simulink model "BaseEngineController_A02_bak2"  (Simulink 7.7)
settings: Solver=FixedStepDiscrete  StartTime=0.0  StopTime=10.0  RelTol=1e-3
PreLoadFcn:
  | try, eval([bdroot '_setup']); catch, eval('model_setup', ''); end
InitFcn:
  | try, eval([bdroot '_setup']); catch, eval('model_setup', ''); end
undefined symbols (not defined in the file or in its folder): ECU555, High, motohawk_build_version, motohawk_trunc_name, motohawk_vardec_config_group, motohawk_vardec_path, True
libraries: MotoHawk_lib x1081, AutoDoc_lib x609, motohawk_control_primitives_lib x132, motohawk_engine_lib x26, motohawk_annotations_lib x9, simulink x8, motohawk_control_primitives_motoshare_lib x1, simulink_extras x1
(part 4 of 22)

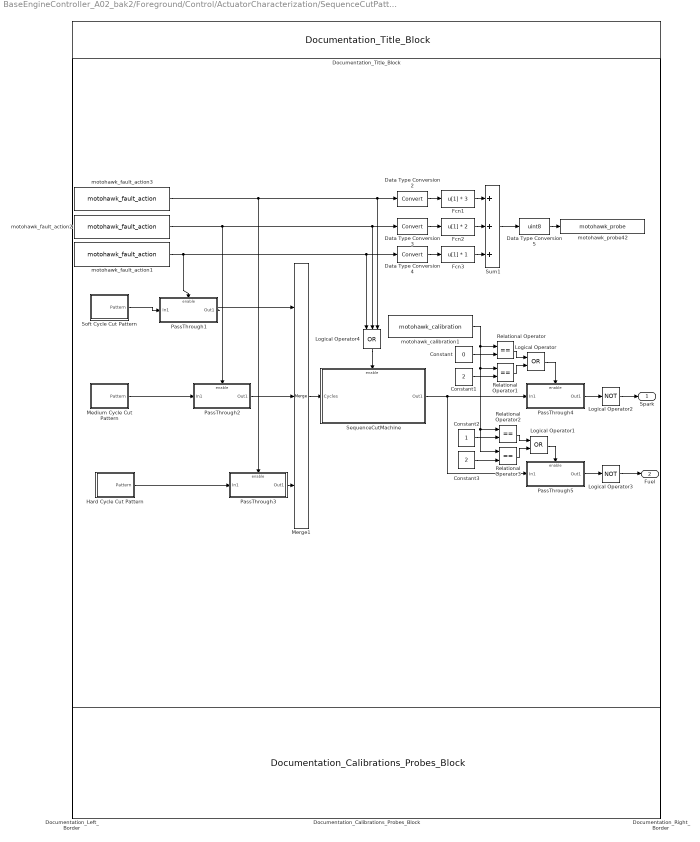
== system BaseEngineController_A02_bak2/Foreground/Control/ActuatorCharacterization/SequenceCutPattern ==
  Constant : Constant  Value=0  OutDataTypeStr=Inherit: Inherit via back propagation  SampleTime=-1
  Constant1 : Constant  Value=2  OutDataTypeStr=Inherit: Inherit via back propagation  SampleTime=-1
  Constant2 : Constant  OutDataTypeStr=Inherit: Inherit via back propagation  SampleTime=-1
  Constant3 : Constant  Value=2  OutDataTypeStr=Inherit: Inherit via back propagation  SampleTime=-1
  Data Type Conversion2 : DataTypeConversion  SaturateOnIntegerOverflow=on  RndMeth=Zero
  Data Type Conversion3 : DataTypeConversion  SaturateOnIntegerOverflow=on  RndMeth=Zero
  Data Type Conversion4 : DataTypeConversion  SaturateOnIntegerOverflow=on  RndMeth=Zero
  Data Type Conversion5 : DataTypeConversion  OutDataTypeStr=uint8  SaturateOnIntegerOverflow=on  RndMeth=Zero
  Documentation_Calibrations_Probes_Block : AutoDoc_lib/Documentation_Calibrations_Probes_Block
  Documentation_Left_Border : AutoDoc_lib/Documentation_Left_Border  layout=Portrait
  Documentation_Right_Border : AutoDoc_lib/Documentation_Right_Border  layout=Portrait
  Documentation_Title_Block : AutoDoc_lib/Documentation_Title_Block
  Fcn1 : Fcn  Expr=u[1] * 3
  Fcn2 : Fcn  Expr=u[1] * 2
  Fcn3 : Fcn  Expr=u[1] * 1
  Hard Cycle Cut Pattern : Subsystem
  Logical Operator : Logic  Operator=OR
  Logical Operator1 : Logic  Operator=OR
  Logical Operator2 : Logic  Operator=NOT
  Logical Operator3 : Logic  Operator=NOT
  Logical Operator4 : Logic  Operator=OR  Inputs=3  AllPortsSameDT=off
  Medium Cycle Cut Pattern : Subsystem
  Merge1 : Merge  Inputs=3  AllowUnequalInputPortWidths=on
  PassThrough1 : Subsystem  TreatAsAtomicUnit=on
  PassThrough2 : Subsystem  TreatAsAtomicUnit=on
  PassThrough3 : Subsystem  TreatAsAtomicUnit=on
  PassThrough4 : Subsystem  TreatAsAtomicUnit=on
  PassThrough5 : Subsystem  TreatAsAtomicUnit=on
  Relational Operator{,1..3} (4 alike) : RelationalOperator  Operator===  InputSameDT=on
  SequenceCutMachine : Subsystem  TreatAsAtomicUnit=on
      mask Description:
      | When enabled, this block cycles though the 4 input patterns of cylinder disables that can be applied to fuel or spark.
      | 
      | The output should act on the enable inputs for MotoHawk EST and FInj blocks.  Outputs are reset when disabled.
      | 
      | Calibration arrays should be cast as boolean, and can be set up for any cylinder configuration.
      mask Display:
      | patch([0 0 1 1], [0 0.075 0.075 0], [230/255 0/255 0/255])
      | patch([0 0 1 1], [0.025 0.05 0.05 0.025], [150/255 0/255 0/255])
      | patch([0 0 1 1], [0 0.025 0.025 0], [0/255 0/255 0/255])
      | 
      | disp('SEQUENCE DISABLE\nPATTERN');
  Soft Cycle Cut Pattern : Subsystem
  Sum1 : Sum  Inputs=+++  SaturateOnIntegerOverflow=on
  motohawk_calibration1 : MotoHawk_lib/Calibration & Probing Blocks/motohawk_calibration  nam='RevLimitType'  val=0  storage=Calibration  show_more=on  name_source=Use Parameter  data_type=int8  read_level=1  write_level=1  ovr_cals=on  view_as=Enumeration  enum_struct={'Cut Spark', 'Cut Fuel', 'Cut Spark and Fuel'}  vector_style=Wide Row  use_help_units=on  help='Define the type of sequence to be disable when in Rev Limit'  units=''  use_header_enum=off  row_header_enum={}  col_header_enum={}  use_min_max=off  min_val=-inf  max_val=inf  use_precision=off  precision=''  gain=1  offset=0  exp=1  use_mototune_group=on  mototune_group=motohawk_vardec_path(gcb)
  motohawk_fault_action1 : MotoHawk_lib/Fault Management Blocks/motohawk_fault_action  nam='SoftRevLimit'  output_status=on  eval_nam=SoftRevLimit
  motohawk_fault_action2 : MotoHawk_lib/Fault Management Blocks/motohawk_fault_action  nam='MediumRevLimit'  output_status=on  eval_nam=MediumRevLimit
  motohawk_fault_action3 : MotoHawk_lib/Fault Management Blocks/motohawk_fault_action  nam='HardRevLimit'  output_status=on  eval_nam=HardRevLimit
  motohawk_probe42 : MotoHawk_lib/Calibration & Probing Blocks/motohawk_probe  nam='RevLimiterState'  show_more=on  name_source=Use Parameter  read_level=1  view_as=Enumeration  enum_struct={'Disabled', 'Soft', 'Medium', 'Hard'}  vector_style=Wide Row  use_help_units=off  help=''  units=''  use_header_enum=off  row_header_enum={}  col_header_enum={}  use_precision=off  precision=''  gain=1  offset=0  exp=1  use_mototune_group=on  mototune_group=motohawk_vardec_path(gcb)
  Spark : Outport
  Fuel : Outport  Port=2
  wires:
  Logical Operator/1 -> PassThrough4/Enable
  PassThrough4/1(Out1) -> Logical Operator2/1
  PassThrough5/1(Out1) -> Logical Operator3/1
  Logical Operator1/1 -> PassThrough5/Enable
  Relational Operator/1 -> Logical Operator/1
  Relational Operator1/1 -> Logical Operator/2
  motohawk_calibration1/1 -> Relational Operator/1, Relational Operator1/1, Relational Operator2/1, Relational Operator3/1
  Constant/1 -> Relational Operator/2
  Constant1/1 -> Relational Operator1/2
  Constant2/1 -> Relational Operator2/2
  Constant3/1 -> Relational Operator3/2
  Relational Operator2/1 -> Logical Operator1/1
  Relational Operator3/1 -> Logical Operator1/2
  Logical Operator2/1 -> Spark/1
  Logical Operator3/1 -> Fuel/1
  SequenceCutMachine/1(Out1) -> PassThrough4/1(In1), PassThrough5/1(In1)
  Merge1/1 -> SequenceCutMachine/1(Cycles)
  PassThrough1/1(Out1) -> Merge1/1
  motohawk_fault_action1/1 -> PassThrough1/Enable, Logical Operator4/1, Data Type Conversion4/1
  Medium Cycle Cut Pattern/1(Pattern) -> PassThrough2/1(In1)
  PassThrough2/1(Out1) -> Merge1/2
  motohawk_fault_action2/1 -> PassThrough2/Enable, Logical Operator4/2, Data Type Conversion3/1
  Hard Cycle Cut Pattern/1(Pattern) -> PassThrough3/1(In1)
  PassThrough3/1(Out1) -> Merge1/3
  motohawk_fault_action3/1 -> PassThrough3/Enable, Logical Operator4/3, Data Type Conversion2/1
  Logical Operator4/1 -> SequenceCutMachine/Enable
  Soft Cycle Cut Pattern/1(Pattern) -> PassThrough1/1(In1)
  Data Type Conversion2/1 -> Fcn1/1
  Data Type Conversion3/1 -> Fcn2/1
  Data Type Conversion4/1 -> Fcn3/1
  Fcn1/1 -> Sum1/1
  Fcn2/1 -> Sum1/2
  Fcn3/1 -> Sum1/3
  Sum1/1 -> Data Type Conversion5/1
  Data Type Conversion5/1 -> motohawk_probe42/1

== system BaseEngineController_A02_bak2/Foreground/Control/ActuatorCharacterization/Spark Advance to Start Angle ==
  Stop Angle [degCA] : Inport
  RPM : Inport  Port=2
  PulseWidth [ms] : Inport  Port=3
  Trigger : TriggerPort  TriggerType=function-call
  Documentation_Calibrations_Probes_Block : AutoDoc_lib/Documentation_Calibrations_Probes_Block
  Documentation_Left_Border : AutoDoc_lib/Documentation_Left_Border  layout=Portrait
  Documentation_Right_Border : AutoDoc_lib/Documentation_Right_Border  layout=Portrait
  Documentation_Title_Block : AutoDoc_lib/Documentation_Title_Block
  Product1 : Product  Inputs=**/  SaturateOnIntegerOverflow=on  InputSameDT=on  OutDataTypeStr=Inherit: Same as first input
  Product2 : Product  Inputs=**  SaturateOnIntegerOverflow=on  InputSameDT=on  OutDataTypeStr=Inherit: Same as first input
  Sum : Sum  Inputs=|++  SaturateOnIntegerOverflow=on  InputSameDT=on
  deg/rev : Constant  Value=360
  min/msec : Constant  Value=60000
  Start Angle [degCA] : Outport
  wires:
  Sum/1 -> Start Angle [degCA]/1
  RPM/1 -> Product1/1
  min/msec/1 -> Product1/3
  deg/rev/1 -> Product1/2
  PulseWidth [ms]/1 -> Product2/2  "PulseWidth [ms]"
  Product1/1 -> Product2/1  "deg/ms"
  Product2/1 -> Sum/2  "PulseWidth [degCA]"
  Stop Angle [degCA]/1 -> Sum/1

== system BaseEngineController_A02_bak2/Foreground/Control/ActuatorCharacterization/Spark Coil Characterization ==
  SparkEnergy [mJ] : Inport
  SysVolt09Idx : Inport  Port=2
  Trigger : TriggerPort  TriggerType=function-call
  Constant : Constant  Value=1000
  Constant1 : Constant  Value=0  OutDataTypeStr=Inherit: Inherit via back propagation
  Documentation_Calibrations_Probes_Block : AutoDoc_lib/Documentation_Calibrations_Probes_Block
  Documentation_Left_Border : AutoDoc_lib/Documentation_Left_Border  layout=Portrait
  Documentation_Right_Border : AutoDoc_lib/Documentation_Right_Border  layout=Portrait
  Documentation_Title_Block : AutoDoc_lib/Documentation_Title_Block
  Only Enable Spark when Energy is Above Zero : RelationalOperator  Operator=>  ZeroCross=off  InputSameDT=on
  Product : Product  Inputs=*/  SaturateOnIntegerOverflow=on  OutDataTypeStr=Inherit: Same as first input
  Sum : Sum  Inputs=|++  SaturateOnIntegerOverflow=on
  motohawk_calibration : MotoHawk_lib/Calibration & Probing Blocks/motohawk_calibration  nam='CoilDrvrDlyTime'  val=0.004  storage=Calibration  show_more=on  name_source=Use Parameter  data_type=Inherit from 'Default Value'  read_level=1  write_level=1  ovr_cals=on  view_as=Number  enum_struct={'Disabled', 'Enabled'}  vector_style=Wide Row  use_help_units=on  help='Coil driver delay time between receipt of EST signal and initiation of coil dwell.'  units='ms'  use_header_enum=off  row_header_enum={}  col_header_enum={}  use_min_max=off  min_val=-inf  max_val=inf  use_precision=off  precision='2.3'  gain=1  offset=0  exp=1  use_mototune_group=on  mototune_group=motohawk_vardec_path(gcb)
  motohawk_interpolation_2d1 : MotoHawk_lib/Lookup Tables/motohawk_interpolation_2d  nam='CoilDwell'  table_data=ones(9)*2  show_more=on  data_type=Inherit from 'Table Data'  storage=NonAdaptive  read_level=1  write_level=1  ovr_cals=on  use_help=on  help='Required spark dwell to give the desired spark energy.'  units='ms'  use_min_max=on  min_val=-inf  max_val=inf  use_precision=on  precision=''  gain=1  offset=0  exp=1  use_links=off  row_prelookup_block=''  col_prelookup_block=''  output_name=''  use_mototune_group=on  mototune_group=motohawk_vardec_path(gcb)
  motohawk_override_rel : MotoHawk_lib/Calibration & Probing Blocks/motohawk_override_rel  SystemSampleTime=-1  parent_name='SparkDwell'  show_more=on  name_source=Use Parameter  parent_read_level=1  parent_override_level=1  use_help_units=off  parent_help=''  parent_units=''  use_min_max=off  parent_min=-inf  parent_max=inf  use_precision=off  parent_precision=''  parent_gain=1  parent_offset=0  parent_exp=1  use_mototune_group=on  parent_group=motohawk_vardec_path(gcb)
  motohawk_prelookup : MotoHawk_lib/Lookup Tables/motohawk_prelookup  nam='SpkEnergy09'  breakpoint_data=[10 19 29 38 48 57 66 76 86]  show_more=on  data_type=Inherit from 'Table Data'  storage=Constant  read_level=1  write_level=1  ovr_cals=on  use_help=on  help=''  units=''  use_min_max=off  min_val=-inf  max_val=inf  use_precision=on  precision=''  gain=1  offset=0  exp=1  use_links=off  input_name=''  use_mototune_group=on  mototune_group=motohawk_vardec_path(gcb)
  motohawk_probe : MotoHawk_lib/Calibration & Probing Blocks/motohawk_probe  nam='SparkDwell'  show_more=on  name_source=Use Parameter  read_level=1  view_as=Number  enum_struct={'Disabled', 'Enabled'}  vector_style=Wide Row  use_help_units=on  help='Total electrical spark dwell time'  units='ms'  use_header_enum=off  row_header_enum={}  col_header_enum={}  use_precision=off  precision=''  gain=1  offset=0  exp=1  use_mototune_group=on  mototune_group=motohawk_vardec_path(gcb)
  motohawk_probe1 : MotoHawk_lib/Calibration & Probing Blocks/motohawk_probe  nam='SparkEnergyMonitor'  show_more=on  name_source=Use Parameter  read_level=1  view_as=Number  enum_struct={'Disabled', 'Enabled'}  vector_style=Wide Row  use_help_units=on  help='Commanded spark energy, before conversion to dwell time'  units='mJ'  use_header_enum=off  row_header_enum={}  col_header_enum={}  use_precision=off  precision=''  gain=1  offset=0  exp=1  use_mototune_group=on  mototune_group=motohawk_vardec_path(gcb)
  motohawk_probe2 : MotoHawk_lib/Calibration & Probing Blocks/motohawk_probe  nam='SparkEnabled'  show_more=on  name_source=Use Parameter  read_level=1  view_as=Number  enum_struct={'Disabled', 'Enabled'}  vector_style=Wide Row  use_help_units=on  help='Enabled when spark energy is greater than zero'  units='boolean'  use_header_enum=off  row_header_enum={}  col_header_enum={}  use_precision=off  precision=''  gain=1  offset=0  exp=1  use_mototune_group=on  mototune_group=motohawk_vardec_path(gcb)
  SparkDwell [ms] : Outport
  SparkEnable [bool] : Outport  Port=2
  wires:
  SysVolt09Idx/1 -> motohawk_interpolation_2d1/2  "SysVolt10Idx"
  motohawk_prelookup/1 -> motohawk_interpolation_2d1/1  "SpkEnergy09Idx"
  SparkEnergy [mJ]/1 -> motohawk_probe1/1, Only Enable Spark when Energy is Above Zero/1, motohawk_prelookup/1
  Sum/1 -> motohawk_override_rel/1
  motohawk_calibration/1 -> Product/1
  Product/1 -> Sum/2
  Constant/1 -> Product/2
  Only Enable Spark when Energy is Above Zero/1 -> SparkEnable [bool]/1, motohawk_probe2/1
  Constant1/1 -> Only Enable Spark when Energy is Above Zero/2
  motohawk_override_rel/1 -> motohawk_probe/1, SparkDwell [ms]/1
  motohawk_interpolation_2d1/1 -> Sum/1

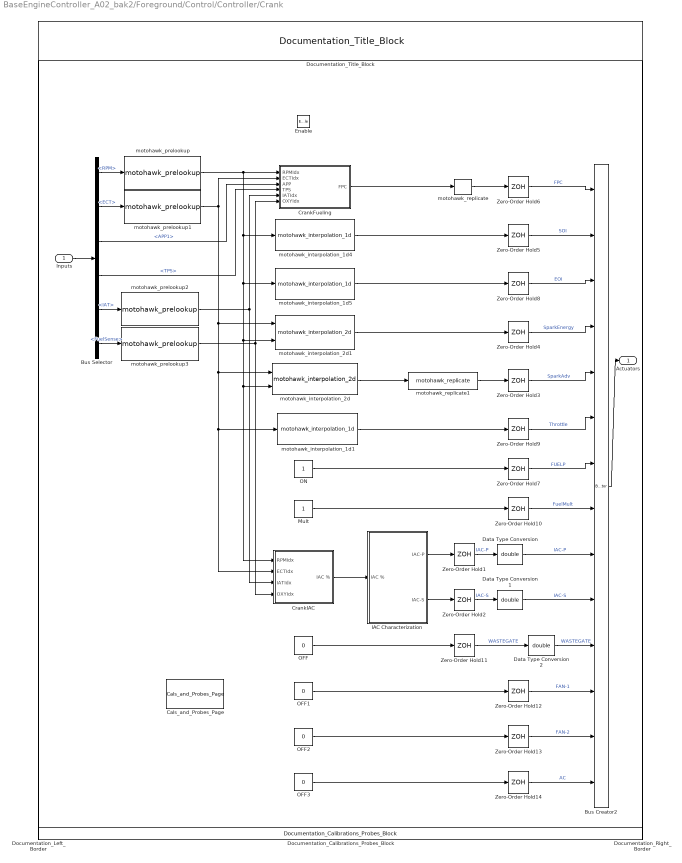
== system BaseEngineController_A02_bak2/Foreground/Control/Controller/Crank ==
  Inputs : Inport
  Enable : EnablePort  StatesWhenEnabling=reset
  Bus Creator2 : BusCreator  Inputs=14
  Bus Selector : BusSelector  OutputSignals=Raw.RPM,Raw.NonLinearSensors.ECT,RawProcessed.APP1,RawProcessed.TPS,Raw.NonLinearSensors.IAT,Raw.LinearSensors.FuelSense
  Cals_and_Probes_Page : AutoDoc_lib/Cals_and_Probes_Page  SystemSampleTime=-1
  CrankFueling : Subsystem
  CrankIAC : Subsystem
  Data Type Conversion : DataTypeConversion  OutDataTypeStr=double
  Data Type Conversion1 : DataTypeConversion  OutDataTypeStr=double
  Data Type Conversion2 : DataTypeConversion  OutDataTypeStr=double
  Documentation_Calibrations_Probes_Block : AutoDoc_lib/Documentation_Calibrations_Probes_Block
  Documentation_Left_Border : AutoDoc_lib/Documentation_Left_Border  layout=Portrait
  Documentation_Right_Border : AutoDoc_lib/Documentation_Right_Border  layout=Portrait
  Documentation_Title_Block : AutoDoc_lib/Documentation_Title_Block
  IAC Characterization : Subsystem
  Mult : Constant  OutDataTypeStr=double
  OFF{,1..3} (4 alike) : Constant  Value=0  OutDataTypeStr=boolean
  ON : Constant  OutDataTypeStr=boolean
  Zero-Order Hold{1..14} (14 alike) : ZeroOrderHold  SampleTime=-1
  motohawk_interpolation_1d1 : MotoHawk_lib/Lookup Tables/motohawk_interpolation_1d  nam='CrankAirflowSetPt'  table_data=[34 34 34 31 30]  show_more=on  data_type=Inherit from 'Table Data'  storage=NonAdaptive  read_level=1  write_level=1  ovr_cals=on  use_help=on  help='ETC setpoint during cranking.'  units='%'  use_min_max=off  min_val=-inf  max_val=inf  use_precision=off  precision=''  gain=1  offset=0  exp=1  use_links=off  prelookup_block=''  output_name=''  use_mototune_group=on  mototune_group=motohawk_vardec_path(gcb)
  motohawk_interpolation_1d4 : MotoHawk_lib/Lookup Tables/motohawk_interpolation_1d  nam='CrankSOI'  table_data=[500 500 500 500 500]  show_more=on  data_type=Inherit from 'Table Data'  storage=NonAdaptive  read_level=1  write_level=1  ovr_cals=on  use_help=on  help='Start of injection during cranking.'  units='degBTDC'  use_min_max=on  min_val=-920  max_val=920  use_precision=on  precision=''  gain=1  offset=0  exp=1  use_links=off  prelookup_block=''  output_name=''  use_mototune_group=on  mototune_group=motohawk_vardec_path(gcb)
  motohawk_interpolation_1d5 : MotoHawk_lib/Lookup Tables/motohawk_interpolation_1d  nam='CrankMakeUpEOI'  table_data=[200 200 200 200 200]  show_more=on  data_type=Inherit from 'Table Data'  storage=NonAdaptive  read_level=1  write_level=1  ovr_cals=on  use_help=on  help='Start of injection during cranking.'  units='degBTDC'  use_min_max=on  min_val=-920  max_val=920  use_precision=on  precision=''  gain=1  offset=0  exp=1  use_links=off  prelookup_block=''  output_name=''  use_mototune_group=on  mototune_group=motohawk_vardec_path(gcb)
  motohawk_interpolation_2d : MotoHawk_lib/Lookup Tables/motohawk_interpolation_2d  nam='CrankSpark'  table_data=ones(5,5)  show_more=on  data_type=Inherit from 'Table Data'  storage=NonAdaptive  read_level=1  write_level=1  ovr_cals=on  use_help=on  help='Spark adv during cranking.'  units='degBTDC'  use_min_max=on  min_val=-100  max_val=100  use_precision=off  precision=''  gain=1  offset=0  exp=1  use_links=off  row_prelookup_block=''  col_prelookup_block=''  output_name=''  use_mototune_group=on  mototune_group=motohawk_vardec_path(gcb)
  motohawk_interpolation_2d1 : MotoHawk_lib/Lookup Tables/motohawk_interpolation_2d  nam='CrankSpkEnergy'  table_data=[80 72.5 65 57 50;80 72.5 65 57.5 50;80 72.5 65 57.5 50;80 72.5 65 57.5 50;80 72.5 65 57.5 50]  show_more=on  data_type=Inherit from 'Table Data'  storage=NonAdaptive  read_level=1  write_level=1  ovr_cals=on  use_help=on  help='Spark energy during cranking.'  units='MJ'  use_min_max=on  min_val=0  max_val=inf  use_precision=on  precision=''  gain=1  offset=0  exp=1  use_links=off  row_prelookup_block=''  col_prelookup_block=''  output_name=''  use_mototune_group=on  mototune_group=motohawk_vardec_path(gcb)
  motohawk_prelookup : MotoHawk_lib/Lookup Tables/motohawk_prelookup  nam='CrankRPM05'  breakpoint_data=[0 100 200 400 500]  show_more=on  data_type=Inherit from 'Table Data'  storage=Constant  read_level=1  write_level=1  ovr_cals=on  use_help=on  help='RPM pre-lookup for crank maps'  units='RPM'  use_min_max=off  min_val=-inf  max_val=inf  use_precision=off  precision=''  gain=1  offset=0  exp=1  use_links=on  input_name=''  use_mototune_group=on  mototune_group=motohawk_vardec_path(gcb)
  motohawk_prelookup1 : MotoHawk_lib/Lookup Tables/motohawk_prelookup  nam='CrankECT05'  breakpoint_data=[-40 -3.75 32.5 68.75 105]  show_more=on  data_type=Inherit from 'Table Data'  storage=Constant  read_level=1  write_level=1  ovr_cals=on  use_help=on  help='ECT pre-lookup for crank maps.'  units='degC'  use_min_max=off  min_val=-inf  max_val=inf  use_precision=off  precision=''  gain=1  offset=0  exp=1  use_links=on  input_name='ECT'  use_mototune_group=on  mototune_group=motohawk_vardec_path(gcb)
  motohawk_prelookup2 : MotoHawk_lib/Lookup Tables/motohawk_prelookup  nam='CrankIAT05'  breakpoint_data=[-40 -3.75 32.5 68.75 105]  show_more=on  data_type=Inherit from 'Table Data'  storage=Constant  read_level=1  write_level=1  ovr_cals=on  use_help=on  help='IAT pre-lookup for crank maps.'  units='degC'  use_min_max=off  min_val=-inf  max_val=inf  use_precision=off  precision=''  gain=1  offset=0  exp=1  use_links=on  input_name='IAT'  use_mototune_group=on  mototune_group=motohawk_vardec_path(gcb)
  motohawk_prelookup3 : MotoHawk_lib/Lookup Tables/motohawk_prelookup  nam='CrankOXY05'  breakpoint_data=[0 25 50 75 100]  show_more=on  data_type=Inherit from 'Table Data'  storage=Constant  read_level=1  write_level=1  ovr_cals=on  use_help=on  help='OXY pre-lookup for crank maps.'  units='%'  use_min_max=off  min_val=-inf  max_val=inf  use_precision=off  precision=''  gain=1  offset=0  exp=1  use_links=off  input_name=''  use_mototune_group=on  mototune_group=motohawk_vardec_path(gcb)
  motohawk_replicate : MotoHawk_lib/Extra Development Blocks/motohawk_replicate  output_width=-1
  motohawk_replicate1 : MotoHawk_lib/Extra Development Blocks/motohawk_replicate  output_width=-1
  Actuators : Outport
  wires:
  Inputs/1 -> Bus Selector/1
  motohawk_prelookup1/1 -> CrankFueling/2(ECTIdx), motohawk_interpolation_2d1/1, motohawk_interpolation_2d/1, motohawk_interpolation_1d1/1, CrankIAC/2(ECTIdx)
  motohawk_prelookup/1 -> motohawk_interpolation_1d4/1, motohawk_interpolation_1d5/1, motohawk_interpolation_2d1/2, motohawk_interpolation_2d/2, CrankIAC/1(RPMIdx), CrankFueling/1(RPMIdx)
  Bus Selector/2 -> motohawk_prelookup1/1  "<ECT>"
  Bus Selector/1 -> motohawk_prelookup/1  "<RPM>"
  CrankFueling/1(FPC) -> motohawk_replicate/1
  Zero-Order Hold6/1 -> Bus Creator2/1  "FPC"
  Zero-Order Hold5/1 -> Bus Creator2/2  "SOI"
  ON/1 -> Zero-Order Hold7/1
  motohawk_interpolation_1d1/1 -> Zero-Order Hold9/1
  motohawk_interpolation_1d4/1 -> Zero-Order Hold5/1
  Zero-Order Hold4/1 -> Bus Creator2/4  "SparkEnergy"
  Zero-Order Hold3/1 -> Bus Creator2/5  "SparkAdv"
  Zero-Order Hold9/1 -> Bus Creator2/6  "Throttle"
  Zero-Order Hold7/1 -> Bus Creator2/7  "FUELP"
  motohawk_replicate/1 -> Zero-Order Hold6/1
  motohawk_interpolation_1d5/1 -> Zero-Order Hold8/1
  Zero-Order Hold8/1 -> Bus Creator2/3  "EOI"
  motohawk_replicate1/1 -> Zero-Order Hold3/1
  Bus Selector/3 -> CrankFueling/3(APP)  "<APP1>"
  Zero-Order Hold10/1 -> Bus Creator2/8  "FuelMult"
  Mult/1 -> Zero-Order Hold10/1
  motohawk_interpolation_2d1/1 -> Zero-Order Hold4/1
  Bus Selector/4 -> CrankFueling/4(TPS)  "<TPS>"
  Bus Creator2/1 -> Actuators/1
  Zero-Order Hold1/1 -> Data Type Conversion/1  "IAC-P"
  Zero-Order Hold2/1 -> Data Type Conversion1/1  "IAC-S"
  OFF/1 -> Zero-Order Hold11/1
  Zero-Order Hold11/1 -> Data Type Conversion2/1  "WASTEGATE"
  Bus Selector/5 -> motohawk_prelookup2/1  "<IAT>"
  motohawk_prelookup2/1 -> CrankFueling/5(IATIdx), CrankIAC/3(IATIdx)
  Bus Selector/6 -> motohawk_prelookup3/1  "<FuelSense>"
  motohawk_prelookup3/1 -> CrankFueling/6(OXYIdx), CrankIAC/4(OXYIdx)
  motohawk_interpolation_2d/1 -> motohawk_replicate1/1
  CrankIAC/1(IAC %) -> IAC Characterization/1(IAC %)
  IAC Characterization/2(IAC-S) -> Zero-Order Hold2/1
  IAC Characterization/1(IAC-P) -> Zero-Order Hold1/1
  OFF1/1 -> Zero-Order Hold12/1
  Zero-Order Hold12/1 -> Bus Creator2/12  "FAN-1"
  OFF2/1 -> Zero-Order Hold13/1
  Zero-Order Hold13/1 -> Bus Creator2/13  "FAN-2"
  OFF3/1 -> Zero-Order Hold14/1
  Zero-Order Hold14/1 -> Bus Creator2/14  "AC"
  Data Type Conversion/1 -> Bus Creator2/9  "IAC-P"
  Data Type Conversion1/1 -> Bus Creator2/10  "IAC-S"
  Data Type Conversion2/1 -> Bus Creator2/11  "WASTEGATE"

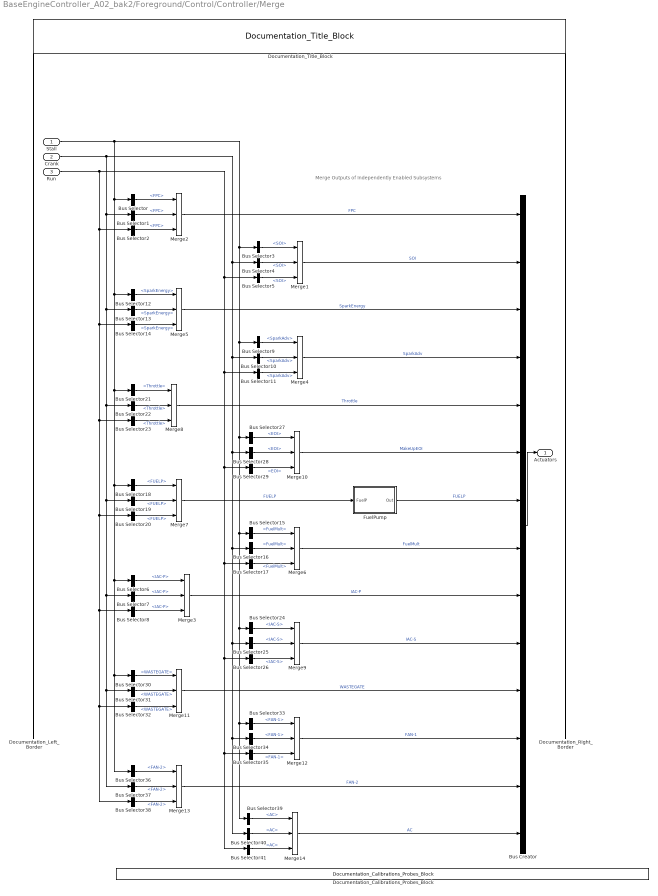
== system BaseEngineController_A02_bak2/Foreground/Control/Controller/Merge ==
  note: Merge Outputs of Independently Enabled Subsystems
  Stall : Inport
  Crank : Inport  Port=2
  Run : Inport  Port=3
  Bus Creator : BusCreator  Inputs=14
  Bus Selector : BusSelector  OutputSignals=FPC
  Bus Selector1 : BusSelector  OutputSignals=FPC
  Bus Selector10 : BusSelector  OutputSignals=SparkAdv
  Bus Selector11 : BusSelector  OutputSignals=SparkAdv
  Bus Selector12 : BusSelector  OutputSignals=SparkEnergy
  Bus Selector13 : BusSelector  OutputSignals=SparkEnergy
  Bus Selector14 : BusSelector  OutputSignals=SparkEnergy
  Bus Selector15 : BusSelector  OutputSignals=FuelMult
  Bus Selector16 : BusSelector  OutputSignals=FuelMult
  Bus Selector17 : BusSelector  OutputSignals=FuelMult
  Bus Selector18 : BusSelector  OutputSignals=FUELP
  Bus Selector19 : BusSelector  OutputSignals=FUELP
  Bus Selector2 : BusSelector  OutputSignals=FPC
  Bus Selector20 : BusSelector  OutputSignals=FUELP
  Bus Selector21 : BusSelector  OutputSignals=Throttle
  Bus Selector22 : BusSelector  OutputSignals=Throttle
  Bus Selector23 : BusSelector  OutputSignals=Throttle
  Bus Selector24 : BusSelector  OutputSignals=IAC-S
  Bus Selector25 : BusSelector  OutputSignals=IAC-S
  Bus Selector26 : BusSelector  OutputSignals=IAC-S
  Bus Selector27 : BusSelector  OutputSignals=EOI
  Bus Selector28 : BusSelector  OutputSignals=EOI
  Bus Selector29 : BusSelector  OutputSignals=EOI
  Bus Selector3 : BusSelector  OutputSignals=SOI
  Bus Selector30 : BusSelector  OutputSignals=WASTEGATE
  Bus Selector31 : BusSelector  OutputSignals=WASTEGATE
  Bus Selector32 : BusSelector  OutputSignals=WASTEGATE
  Bus Selector33 : BusSelector  OutputSignals=FAN-1
  Bus Selector34 : BusSelector  OutputSignals=FAN-1
  Bus Selector35 : BusSelector  OutputSignals=FAN-1
  Bus Selector36 : BusSelector  OutputSignals=FAN-2
  Bus Selector37 : BusSelector  OutputSignals=FAN-2
  Bus Selector38 : BusSelector  OutputSignals=FAN-2
  Bus Selector39 : BusSelector  OutputSignals=AC
  Bus Selector4 : BusSelector  OutputSignals=SOI
  Bus Selector40 : BusSelector  OutputSignals=AC
  Bus Selector41 : BusSelector  OutputSignals=AC
  Bus Selector5 : BusSelector  OutputSignals=SOI
  Bus Selector6 : BusSelector  OutputSignals=IAC-P
  Bus Selector7 : BusSelector  OutputSignals=IAC-P
  Bus Selector8 : BusSelector  OutputSignals=IAC-P
  Bus Selector9 : BusSelector  OutputSignals=SparkAdv
  Documentation_Calibrations_Probes_Block : AutoDoc_lib/Documentation_Calibrations_Probes_Block
  Documentation_Left_Border : AutoDoc_lib/Documentation_Left_Border  layout=Portrait
  Documentation_Right_Border : AutoDoc_lib/Documentation_Right_Border  layout=Portrait
  Documentation_Title_Block : AutoDoc_lib/Documentation_Title_Block
  FuelPump : Subsystem
  Merge{1..14} (14 alike) : Merge  Inputs=3
  Actuators : Outport
  wires:
  Bus Selector23/1 -> Merge8/3  "<Throttle>"
  Bus Selector22/1 -> Merge8/2  "<Throttle>"
  Bus Selector21/1 -> Merge8/1  "<Throttle>"
  Bus Selector20/1 -> Merge7/3  "<FUELP>"
  Bus Selector19/1 -> Merge7/2  "<FUELP>"
  Bus Selector18/1 -> Merge7/1  "<FUELP>"
  Merge7/1 -> FuelPump/1(FuelP)  "FUELP"
  Bus Selector14/1 -> Merge5/3  "<SparkEnergy>"
  Bus Selector13/1 -> Merge5/2  "<SparkEnergy>"
  Bus Selector12/1 -> Merge5/1  "<SparkEnergy>"
  Bus Selector11/1 -> Merge4/3  "<SparkAdv>"
  Bus Selector10/1 -> Merge4/2  "<SparkAdv>"
  Bus Selector9/1 -> Merge4/1  "<SparkAdv>"
  Bus Selector5/1 -> Merge1/3  "<SOI>"
  Bus Selector4/1 -> Merge1/2  "<SOI>"
  Bus Selector3/1 -> Merge1/1  "<SOI>"
  Run/1 -> Bus Selector11/1, Bus Selector29/1, Bus Selector17/1, Bus Selector26/1, Bus Selector35/1, Bus Selector41/1, Bus Selector5/1, Bus Selector2/1, Bus Selector14/1, Bus Selector23/1, Bus Selector20/1, Bus Selector8/1, Bus Selector32/1, Bus Selector38/1
  Crank/1 -> Bus Selector10/1, Bus Selector28/1, Bus Selector16/1, Bus Selector25/1, Bus Selector34/1, Bus Selector40/1, Bus Selector4/1, Bus Selector1/1, Bus Selector13/1, Bus Selector22/1, Bus Selector19/1, Bus Selector7/1, Bus Selector31/1, Bus Selector37/1
  Stall/1 -> Bus Selector9/1, Bus Selector27/1, Bus Selector15/1, Bus Selector24/1, Bus Selector33/1, Bus Selector39/1, Bus Selector3/1, Bus Selector/1, Bus Selector12/1, Bus Selector21/1, Bus Selector18/1, Bus Selector6/1, Bus Selector30/1, Bus Selector36/1
  Bus Creator/1 -> Actuators/1
  Bus Selector/1 -> Merge2/1  "<FPC>"
  Bus Selector1/1 -> Merge2/2  "<FPC>"
  Bus Selector2/1 -> Merge2/3  "<FPC>"
  Merge2/1 -> Bus Creator/1  "FPC"
  Merge1/1 -> Bus Creator/2  "SOI"
  Merge5/1 -> Bus Creator/3  "SparkEnergy"
  Merge8/1 -> Bus Creator/5  "Throttle"
  Merge4/1 -> Bus Creator/4  "SparkAdv"
  FuelPump/1(Out) -> Bus Creator/7  "FUELP"
  Bus Selector17/1 -> Merge6/3  "<FuelMult>"
  Bus Selector16/1 -> Merge6/2  "<FuelMult>"
  Bus Selector15/1 -> Merge6/1  "<FuelMult>"
  Merge6/1 -> Bus Creator/8  "FuelMult"
  Bus Selector29/1 -> Merge10/3  "<EOI>"
  Bus Selector28/1 -> Merge10/2  "<EOI>"
  Bus Selector27/1 -> Merge10/1  "<EOI>"
  Merge10/1 -> Bus Creator/6  "MakeUpEOI"
  Bus Selector8/1 -> Merge3/3  "<IAC-P>"
  Bus Selector7/1 -> Merge3/2  "<IAC-P>"
  Bus Selector6/1 -> Merge3/1  "<IAC-P>"
  Merge3/1 -> Bus Creator/9  "IAC-P"
  Bus Selector26/1 -> Merge9/3  "<IAC-S>"
  Bus Selector25/1 -> Merge9/2  "<IAC-S>"
  Bus Selector24/1 -> Merge9/1  "<IAC-S>"
  Merge9/1 -> Bus Creator/10  "IAC-S"
  Bus Selector32/1 -> Merge11/3  "<WASTEGATE>"
  Bus Selector31/1 -> Merge11/2  "<WASTEGATE>"
  Bus Selector30/1 -> Merge11/1  "<WASTEGATE>"
  Merge11/1 -> Bus Creator/11  "WASTEGATE"
  Bus Selector35/1 -> Merge12/3  "<FAN-1>"
  Bus Selector34/1 -> Merge12/2  "<FAN-1>"
  Bus Selector33/1 -> Merge12/1  "<FAN-1>"
  Merge12/1 -> Bus Creator/12  "FAN-1"
  Bus Selector38/1 -> Merge13/3  "<FAN-2>"
  Bus Selector37/1 -> Merge13/2  "<FAN-2>"
  Bus Selector36/1 -> Merge13/1  "<FAN-2>"
  Merge13/1 -> Bus Creator/13  "FAN-2"
  Bus Selector41/1 -> Merge14/3  "<AC>"
  Bus Selector40/1 -> Merge14/2  "<AC>"
  Bus Selector39/1 -> Merge14/1  "<AC>"
  Merge14/1 -> Bus Creator/14  "AC"

== system BaseEngineController_A02_bak2/Foreground/Control/Controller/Run ==
  Inputs : Inport
  Enable : EnablePort  StatesWhenEnabling=reset  ShowOutputPort=on
  AC Control : Subsystem
  AirFlow Manager : Subsystem
  Base Equiv Ratio : Subsystem  TreatAsAtomicUnit=on
  Boost Control : Subsystem
  Bus Creator2 : BusCreator  Inputs=14
  DFCO : Subsystem
  Data Type Conversion : DataTypeConversion  OutDataTypeStr=boolean
  Data Type Conversion1 : DataTypeConversion  OutDataTypeStr=boolean
  Demux : Demux  Outputs=3
  FAN Control : Subsystem
  Fuel System Manager : Subsystem  TreatAsAtomicUnit=on
  IAC Characterization : Subsystem
  MaxGovernor : Subsystem  TreatAsAtomicUnit=on
  MinGovernor : Subsystem  TreatAsAtomicUnit=on
  O2 Control : Subsystem  TreatAsAtomicUnit=on
  ON1 : Constant  OutDataTypeStr=boolean
  Spark Manager : Subsystem  TreatAsAtomicUnit=on
  Zero-Order Hold{1..8,11..12,16..18} (13 alike) : ZeroOrderHold  SampleTime=-1
  motohawk_replicate1 : MotoHawk_lib/Extra Development Blocks/motohawk_replicate  output_width=8
  motohawk_trigger1 : MotoHawk_lib/Trigger Blocks/motohawk_trigger  Tag=Trigger  trigger=FGND_5XRTI_PERIODIC  function_name=Trigger_FGND_5XRTI_PERIODIC_5374p0016  priority_order=1  inherited=on  run_disabled=off  override_app_monitor=off  target=ECU555_80
  Actuators : Outport
  wires:
  Enable/1 -> O2 Control/1(Reset)
  Inputs/1 -> Base Equiv Ratio/1(Sensors), Spark Manager/2(Sensors), MinGovernor/1(Sensors), MaxGovernor/2(Sensors), AirFlow Manager/3(Sensors), Boost Control/1(Sensors), FAN Control/1(Sensors), AC Control/1(Sensors), DFCO/3(Sensors), O2 Control/2(Sensors), Fuel System Manager/1(Sensors)
  ON1/1 -> Zero-Order Hold16/1  "FUELP"
  Bus Creator2/1 -> Actuators/1
  Spark Manager/1(SparkEnergy) -> Zero-Order Hold1/1  "SparkEnergy"
  MinGovernor/1(MinGovInfo) -> Spark Manager/3(RPM_Ctrl_Ofst), AirFlow Manager/1(MinGovInfo), IAC Characterization/1(MinGovINFO), MaxGovernor/1(MinGovInfo), DFCO/2(MinGovInfo)
  Spark Manager/2(SpkAdv) -> Zero-Order Hold12/1  "SparkAdv"
  MaxGovernor/1(MaxGovInfo) -> AirFlow Manager/2(MaxGovInfo)
  Fuel System Manager/1(DesiredFuel) -> motohawk_replicate1/1
  Fuel System Manager/2(InjTiming) -> Zero-Order Hold17/1  "SOI"
  Fuel System Manager/3(EOI) -> Zero-Order Hold3/1  "EOI"
  O2 Control/1(O2_Info) -> Fuel System Manager/2(O2_Info)
  motohawk_replicate1/1 -> Bus Creator2/1  "FPC"
  Fuel System Manager/4(FuelMult) -> Zero-Order Hold4/1  "FuelMult"
  Zero-Order Hold1/1 -> Bus Creator2/5  "SparkEnergy"
  Zero-Order Hold12/1 -> Bus Creator2/6  "SparkAdv"
  Zero-Order Hold16/1 -> Bus Creator2/8  "FUELP"
  Zero-Order Hold18/1 -> Bus Creator2/7  "Throttle"
  Zero-Order Hold3/1 -> Bus Creator2/3  "EOI"
  Zero-Order Hold4/1 -> Bus Creator2/4  "FuelMult"
  Zero-Order Hold17/1 -> Bus Creator2/2  "SOI"
  Demux/1 -> MinGovernor/Trigger
  Demux/2 -> MaxGovernor/Trigger
  Demux/3 -> Spark Manager/Trigger
  motohawk_trigger1/1 -> Demux/1
  Zero-Order Hold2/1 -> Bus Creator2/9  "IAC-P"
  Zero-Order Hold11/1 -> Bus Creator2/11  "WASTEGATE"
  Zero-Order Hold5/1 -> Bus Creator2/10  "IAC-S"
  IAC Characterization/2(IAC-S) -> Zero-Order Hold5/1
  IAC Characterization/1(IAC-P) -> Zero-Order Hold2/1
  Boost Control/1(Wastegate) -> Zero-Order Hold11/1
  Zero-Order Hold6/1 -> Data Type Conversion/1  "FAN-2"
  Zero-Order Hold7/1 -> Data Type Conversion1/1  "FAN-1"
  Zero-Order Hold8/1 -> Bus Creator2/14  "AC"
  FAN Control/1(FAN-1) -> Zero-Order Hold7/1
  FAN Control/2(FAN-2) -> Zero-Order Hold6/1
  AC Control/1(AC) -> Zero-Order Hold8/1, MinGovernor/2(AC)
  Data Type Conversion/1 -> Bus Creator2/13  "FAN-2"
  Data Type Conversion1/1 -> Bus Creator2/12  "FAN-1"
  AirFlow Manager/1(ETC%) -> Zero-Order Hold18/1  "Throttle"
  Base Equiv Ratio/1(EquivRatioInfo) -> DFCO/1(EquivRatioInfo)
  DFCO/1(DesEquivRatio) -> O2 Control/3(Desired_Equiv), Spark Manager/1(DesEquivRatio)
  DFCO/2(DFCOInfo) -> Spark Manager/4(DFCOInfo)

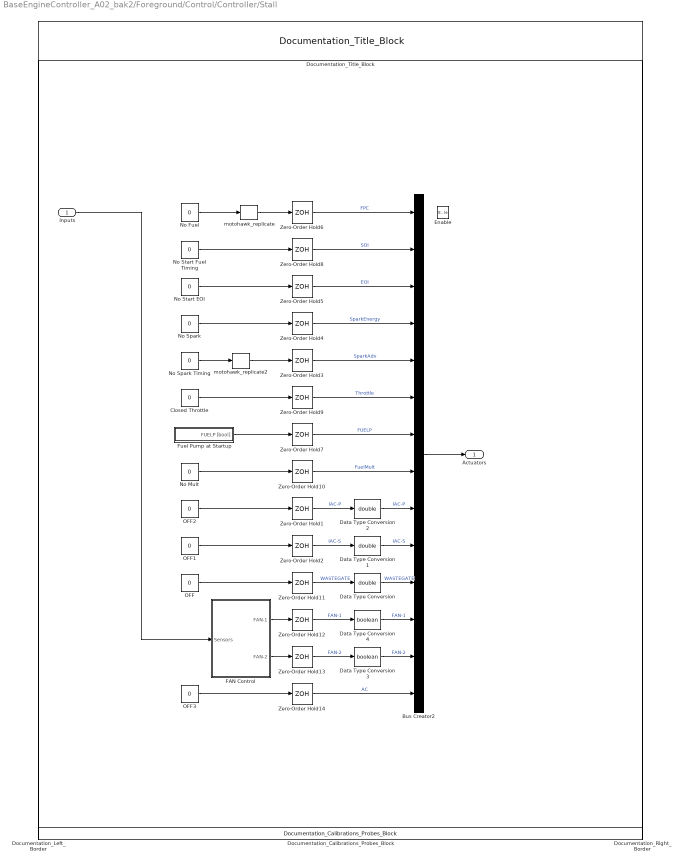
== system BaseEngineController_A02_bak2/Foreground/Control/Controller/Stall ==
  Inputs : Inport
  Enable : EnablePort  StatesWhenEnabling=reset
  Bus Creator2 : BusCreator  Inputs=14
  Closed Throttle : Constant  Value=0
  Data Type Conversion : DataTypeConversion  OutDataTypeStr=double
  Data Type Conversion1 : DataTypeConversion  OutDataTypeStr=double
  Data Type Conversion2 : DataTypeConversion  OutDataTypeStr=double
  Data Type Conversion3 : DataTypeConversion  OutDataTypeStr=boolean
  Data Type Conversion4 : DataTypeConversion  OutDataTypeStr=boolean
  Documentation_Calibrations_Probes_Block : AutoDoc_lib/Documentation_Calibrations_Probes_Block
  Documentation_Left_Border : AutoDoc_lib/Documentation_Left_Border  layout=Portrait
  Documentation_Right_Border : AutoDoc_lib/Documentation_Right_Border  layout=Portrait
  Documentation_Title_Block : AutoDoc_lib/Documentation_Title_Block
  FAN Control : Subsystem
  Fuel Pump at Startup : Subsystem
  No Fuel : Constant  Value=0
  No Mult : Constant  Value=0  OutDataTypeStr=double
  No Spark : Constant  Value=0
  No Spark Timing : Constant  Value=0
  No Start EOI : Constant  Value=0
  No Start Fuel Timing : Constant  Value=0
  OFF{,1..3} (4 alike) : Constant  Value=0  OutDataTypeStr=boolean
  Zero-Order Hold{1..14} (14 alike) : ZeroOrderHold  SampleTime=-1
  motohawk_replicate : MotoHawk_lib/Extra Development Blocks/motohawk_replicate  output_width=-1
  motohawk_replicate2 : MotoHawk_lib/Extra Development Blocks/motohawk_replicate  output_width=-1
  Actuators : Outport
  wires:
  Zero-Order Hold6/1 -> Bus Creator2/1  "FPC"
  No Spark/1 -> Zero-Order Hold4/1
  No Spark Timing/1 -> motohawk_replicate2/1
  No Fuel/1 -> motohawk_replicate/1
  Zero-Order Hold8/1 -> Bus Creator2/2  "SOI"
  No Start Fuel Timing/1 -> Zero-Order Hold8/1
  Bus Creator2/1 -> Actuators/1
  Closed Throttle/1 -> Zero-Order Hold9/1
  Zero-Order Hold4/1 -> Bus Creator2/4  "SparkEnergy"
  Zero-Order Hold3/1 -> Bus Creator2/5  "SparkAdv"
  Zero-Order Hold9/1 -> Bus Creator2/6  "Throttle"
  Zero-Order Hold7/1 -> Bus Creator2/7  "FUELP"
  motohawk_replicate/1 -> Zero-Order Hold6/1
  Zero-Order Hold5/1 -> Bus Creator2/3  "EOI"
  No Start EOI/1 -> Zero-Order Hold5/1
  motohawk_replicate2/1 -> Zero-Order Hold3/1
  No Mult/1 -> Zero-Order Hold10/1
  Zero-Order Hold10/1 -> Bus Creator2/8  "FuelMult"
  Fuel Pump at Startup/1(FUELP [bool]) -> Zero-Order Hold7/1
  OFF2/1 -> Zero-Order Hold1/1
  Zero-Order Hold1/1 -> Data Type Conversion2/1  "IAC-P"
  Zero-Order Hold2/1 -> Data Type Conversion1/1  "IAC-S"
  OFF/1 -> Zero-Order Hold11/1
  Zero-Order Hold11/1 -> Data Type Conversion/1  "WASTEGATE"
  OFF1/1 -> Zero-Order Hold2/1
  Zero-Order Hold12/1 -> Data Type Conversion4/1  "FAN-1"
  Zero-Order Hold13/1 -> Data Type Conversion3/1  "FAN-2"
  Zero-Order Hold14/1 -> Bus Creator2/14  "AC"
  FAN Control/1(FAN-1) -> Zero-Order Hold12/1
  FAN Control/2(FAN-2) -> Zero-Order Hold13/1
  Inputs/1 -> FAN Control/1(Sensors)
  OFF3/1 -> Zero-Order Hold14/1
  Data Type Conversion/1 -> Bus Creator2/11  "WASTEGATE"
  Data Type Conversion1/1 -> Bus Creator2/10  "IAC-S"
  Data Type Conversion2/1 -> Bus Creator2/9  "IAC-P"
  Data Type Conversion3/1 -> Bus Creator2/13  "FAN-2"
  Data Type Conversion4/1 -> Bus Creator2/12  "FAN-1"

== system BaseEngineController_A02_bak2/Foreground/Control/Diagnostics/AC Fault ==
  Sensors : Inport
  Controller : Inport  Port=2
  Bus Selector1 : BusSelector  OutputSignals=Raw.LinearSensors.ACPres
  Bus Selector2 : BusSelector  OutputSignals=AC
  Logical Operator : Logic  AllPortsSameDT=off  OutDataTypeStr=boolean
  Logical Operator1 : Logic  Inputs=3  AllPortsSameDT=off  OutDataTypeStr=boolean
  Logical Operator2 : Logic  AllPortsSameDT=off  OutDataTypeStr=boolean
  Relational Operator : RelationalOperator  OutDataTypeStr=boolean
  Relational Operator1 : RelationalOperator  Operator=<=  OutDataTypeStr=boolean
  Relational Operator3 : RelationalOperator  Operator=>  OutDataTypeStr=boolean
  Relational Operator5 : RelationalOperator  Operator=>  OutDataTypeStr=boolean
  Run State : motohawk_control_primitives_lib/CompareTo  SystemSampleTime=-1  op===  val=3
  Time Since Enabled (With Input)1 : motohawk_control_primitives_lib/Time Since Enabled (With Input)1  SystemSampleTime=-1  nam='ACLowPressureTimer'  samp_time=-1  init=0  upperlim=16000
  Time Since Enabled (With Input)2 : motohawk_control_primitives_lib/Time Since Enabled (With Input)1  SystemSampleTime=-1  nam='AChighPressureTimer'  samp_time=-1  init=0  upperlim=16000
  motohawk_calibration : MotoHawk_lib/Calibration & Probing Blocks/motohawk_calibration  nam='ACHighPressureDisable'  val=3000  storage=Calibration  show_more=on  name_source=Use Parameter  data_type=Inherit from 'Default Value'  read_level=1  write_level=1  ovr_cals=on  view_as=Number  enum_struct={'Disabled', 'Enabled'}  vector_style=Wide Row  use_help_units=on  help='Pressure when ac gets disabled'  units='kpa'  use_header_enum=off  row_header_enum={}  col_header_enum={}  use_min_max=off  min_val=-inf  max_val=inf  use_precision=off  precision=''  gain=1  offset=0  exp=1  use_mototune_group=off  mototune_group=motohawk_vardec_path(gcb)
  motohawk_calibration1 : MotoHawk_lib/Calibration & Probing Blocks/motohawk_calibration  nam='ACLowPressureTime'  val=1  storage=Calibration  show_more=on  name_source=Use Parameter  data_type=double  read_level=1  write_level=1  ovr_cals=on  view_as=Number  enum_struct={'Disabled', 'Enabled'}  vector_style=Wide Row  use_help_units=on  help='After low pressure level reached for this time ACChargeLow_Fault becomes active'  units='s'  use_header_enum=off  row_header_enum={}  col_header_enum={}  use_min_max=off  min_val=0  max_val=inf  use_precision=off  precision=''  gain=1  offset=0  exp=1  use_mototune_group=on  mototune_group=motohawk_vardec_path(gcb)
  motohawk_calibration2 : MotoHawk_lib/Calibration & Probing Blocks/motohawk_calibration  nam='ACLowPressureDisable'  val=300  storage=Calibration  show_more=on  name_source=Use Parameter  data_type=Inherit from 'Default Value'  read_level=1  write_level=1  ovr_cals=on  view_as=Number  enum_struct={'Disabled', 'Enabled'}  vector_style=Wide Row  use_help_units=on  help='Pressure when ac gets disabled'  units='kpa'  use_header_enum=off  row_header_enum={}  col_header_enum={}  use_min_max=off  min_val=-inf  max_val=inf  use_precision=off  precision=''  gain=1  offset=0  exp=1  use_mototune_group=off  mototune_group=motohawk_vardec_path(gcb)
  motohawk_calibration3 : MotoHawk_lib/Calibration & Probing Blocks/motohawk_calibration  nam='ACHighPressureTime'  val=1  storage=Calibration  show_more=on  name_source=Use Parameter  data_type=double  read_level=1  write_level=1  ovr_cals=on  view_as=Number  enum_struct={'Disabled', 'Enabled'}  vector_style=Wide Row  use_help_units=on  help='After high pressure level reached for this time ACChargeHigh_Fault becomes active'  units='s'  use_header_enum=off  row_header_enum={}  col_header_enum={}  use_min_max=off  min_val=0  max_val=inf  use_precision=off  precision=''  gain=1  offset=0  exp=1  use_mototune_group=on  mototune_group=motohawk_vardec_path(gcb)
  motohawk_data_read : MotoHawk_lib/Data Storage Blocks/motohawk_data_read  source=Lookup By Name  structure=Scalar  operation=Read entire data structure at once  sz_vec=4  sz_row=2  sz_col=3  typ=uint8  nam='EngineState'  checkNull=on  struct_inst_name='Struct1'  struct_nam='MyStruct'
  motohawk_fault_def1 : MotoHawk_lib/Fault Management Blocks/motohawk_fault_def  nam='ACChargeHigh_Fault'  mode=Enabled  id=[]  x=25  y=50  input_suspected=on  indeterminate=off  increment_xy=on  downsample_count=1  ovr_cals=on  ovr_actions=on  show_actions=on  action1_name='DisableAC'  action1_cond=Active  action2_name=''  action2_cond=(None)  action3_name=''  action3_cond=(None)  action4_name=''  action4_cond=(None)
  motohawk_fault_def2 : MotoHawk_lib/Fault Management Blocks/motohawk_fault_def  nam='ACChargeLow_Fault'  mode=Enabled  id=[]  x=25  y=50  input_suspected=on  indeterminate=off  increment_xy=on  downsample_count=1  ovr_cals=on  ovr_actions=on  show_actions=on  action1_name='DisableAC'  action1_cond=Active  action2_name=''  action2_cond=(None)  action3_name=''  action3_cond=(None)  action4_name=''  action4_cond=(None)
  wires:
  Sensors/1 -> Bus Selector1/1
  motohawk_data_read/1 -> Run State/1
  Run State/1 -> Logical Operator/1, Logical Operator1/1
  Logical Operator/1 -> Time Since Enabled (With Input)2/1
  Logical Operator1/1 -> Time Since Enabled (With Input)1/1
  motohawk_calibration3/1 -> Relational Operator5/2
  Time Since Enabled (With Input)2/1 -> Relational Operator5/1
  motohawk_calibration1/1 -> Relational Operator3/2
  Time Since Enabled (With Input)1/1 -> Relational Operator3/1
  Relational Operator5/1 -> motohawk_fault_def1/1
  Controller/1 -> Bus Selector2/1
  Relational Operator/1 -> Logical Operator/2
  Bus Selector1/1 -> Relational Operator/1, Relational Operator1/1  "<ACPres>"
  motohawk_calibration/1 -> Relational Operator/2
  motohawk_calibration2/1 -> Relational Operator1/2
  Relational Operator1/1 -> Logical Operator1/2
  Bus Selector2/1 -> Logical Operator1/3, Logical Operator2/2  "<AC>"
  Relational Operator3/1 -> Logical Operator2/1
  Logical Operator2/1 -> motohawk_fault_def2/1
